simulink model "TosAeb"  (R2021a)
settings: Solver=FixedStepDiscrete  StartTime=0.0  StopTime=inf  FixedStep=0.01  RelTol=1e-3
undefined symbols (not defined in the file or in its folder): ACCModSts, AccSts1, AEB_ObjectMovingOrientation, AEB_ObjectMovingState, CN_BTCActive, CN_CurrentGearR, CN_CurrentGearR_DHT, CN_PTCActive, CN_VDCActive, CN_VSC_EspOff, CN_VSC_FCW_AEB_Error, CN_VSC_FCW_AEB_NoDispay, CN_VSC_FCW_AEB_PerfmDgdtn, CN_VSC_FCW_AEB_TempUnAvlbl, CN_VSC_VehStandstill, CNaccelAvoidMax, CNaccelAvoidMin, CNbrakePedalPress, CNcomfortStrLeftAccelMax, CNcomfortStrLeftAccelMin, CNcomfortStrRightAccelMax, CNcomfortStrRightAccelMin, CNDrvDoorOpen, CNDrvModeEco, CNDrvModeNorm, CNDrvModeOther, CNDrvModeSport, CNDrvSBRreminder, CNNull, CNobjInvalidID, CNPassDoorOpen, CNPassSBRreminder, CNPEBSAccelNegInf, CNPEBSAccelNull, CNPEBSTimeInf, CNPEBSTimeNull, CNSTOPMAX, CNSTOPNULL, CNsubjWidth, CNSwtOFF, CNSwtON, CNTTCMAX, CNTTCNULL, CNVehConfigAEBCycNone, CNVehConfigAEBNone, CNVehConfigAEBPedNone, CNVehConfigFCWNone, CNVehConfigJANone, CollisionSeverity, FALSE, FCWSnvty, JACycObjCalcInfoDefaultParams, JAPedObjCalcInfoDefaultParams, JAVehObjCalcInfoDefaultParams, latCycObjCalcInfoDefaultParams, latPedObjCalcInfoDefaultParams, longObjCalcInfoDefaultParams, ModSts, movingStatus, ObjectType ... (+1112)
libraries: simulink x3031, AEBSFunctionLib x50, Ta_Library x33, AEBSStateMachineLib x23, Mylibrary x12, simulink_extras x7, ObjSelect_lib x3, dspstat3 x2
(part 25 of 102)

== system TosAeb/FcwAebJa_Step_sys/ALG_AEBSystem/ALG_AEBController/AEB_FuncCondCalcApp/PedFunctionCondCalc/pedAEBfullSuppAborCond/pedAEBfullAborCondNoStandstillCalc ==
  inputSignals : Inport
  Bus Selector : BusSelector  OutputSignals=latPedTgtCalcInfo.latPedObjCalcInfo.latPedObjRawInfo.ObjectID,latPedTgtCalcInfo.latPedObjCalcInfo.latPedObjRawInfo.ObjectTypeCameraConfirmed,latPedTgtCalcInfo.latPedObjCalcInfo.CollisionRiskProb
  Bus Selector1 : BusSelector  OutputSignals=vehCanInfo.SteerWheelAngle,vehCanInfo.SteerWheelSpd,vehStateCalcInfo.VSCPedFuncSuppAborCond.VSCPedAEBfullAborCond,latFuncActiveStateTime.pedAEBfullWorkTime,vehStateCalcInfo.VSCVehStandStill
  Bus Selector2 : BusSelector  OutputSignals=latPedTgtCalcInfo.latPedCollsionAssesInfo.latCollisionSeverity,latPedTgtCalcInfo.latPedObjCalcInfo.CollisionRiskProb,latPedTgtCalcInfo.latPedObjCalcInfo.latPedObjRawInfo.dy,latPedTgtCalcInfo.latPedObjCalcInfo.latPedObjRawInfo.vy,latPedTgtCalcInfo.latPedObjCalcInfo.latPedObjRawInfo.dx,subjInfo.subjYawRate
  Ground : Ground
  pedAEBfullAborCondFinalJudge : Subsystem
  pedAEBfullObjRelActiveCondCalc : Subsystem
  pedAEBfullSituationRelAborCondCalc : Subsystem
  pedAEBfullVehRelAborCondCalc : Subsystem
  pedAEBfullAborCondNoStandstillFulfilled : Outport
  wires:
  Bus Selector2/6 -> pedAEBfullSituationRelAborCondCalc/7(subjYawRate)  "<subjYawRate>"
  Bus Selector2/5 -> pedAEBfullSituationRelAborCondCalc/6(dx)  "<dx>"
  Bus Selector/3 -> pedAEBfullObjRelActiveCondCalc/3(latCollsionRiskProb)  "<CollisionRiskProb>"
  Bus Selector/2 -> pedAEBfullObjRelActiveCondCalc/2(objIsFusionTarget)  "<ObjectTypeCameraConfirmed>"
  Bus Selector/1 -> pedAEBfullObjRelActiveCondCalc/1(ObjectID)  "<ObjectID>"
  Bus Selector2/4 -> pedAEBfullSituationRelAborCondCalc/5(vy)  "<vy>"
  Bus Selector2/3 -> pedAEBfullSituationRelAborCondCalc/4(dy)  "<dy>"
  Bus Selector2/2 -> pedAEBfullSituationRelAborCondCalc/3(objLatCollsionRiskProb)  "<CollisionRiskProb>"
  Bus Selector2/1 -> pedAEBfullSituationRelAborCondCalc/2(pedCollisionSeverity)  "<latCollisionSeverity>"
  Ground/1 -> pedAEBfullSituationRelAborCondCalc/1(pedVehVelReduced)
  Bus Selector1/5 -> pedAEBfullVehRelAborCondCalc/5(vehStateCalcStandstill)  "<VSCVehStandStill>"
  Bus Selector1/4 -> pedAEBfullVehRelAborCondCalc/4(pedAEBfullWorkTime)  "<pedAEBfullWorkTime>"
  Bus Selector1/3 -> pedAEBfullVehRelAborCondCalc/3(vehStateCalcAEBfullAborFlg)  "<VSCPedAEBfullAborCond>"
  Bus Selector1/2 -> pedAEBfullVehRelAborCondCalc/2(SteerWheelSpd)  "<SteerWheelSpd>"
  Bus Selector1/1 -> pedAEBfullVehRelAborCondCalc/1(SteerWheelAngle)  "<SteerWheelAngle>"
  inputSignals/1 -> Bus Selector1/1, Bus Selector2/1, Bus Selector/1
  pedAEBfullAborCondFinalJudge/1(pedAEBfullAborCondNoStandstillFulfilled) -> pedAEBfullAborCondNoStandstillFulfilled/1
  pedAEBfullSituationRelAborCondCalc/1(pedAEBfullSituationRelAborCondFulfilled) -> pedAEBfullAborCondFinalJudge/3(pedAEBfullSituationRelAborCondFulfilled)  "AEB_pedAEBfullSitRelAborCond"
  pedAEBfullVehRelAborCondCalc/1(pedAEBfullVehRelAborCondFulfilled) -> pedAEBfullAborCondFinalJudge/2(pedAEBfullVehRelAborCondFulfilled)  "AEB_pedAEBfullVehRelAborCond"
  pedAEBfullObjRelActiveCondCalc/1(pedAEBfullObjRelAborCondFulfilled) -> pedAEBfullAborCondFinalJudge/1(pedAEBfullObjRelAborCondFulfilled)  "AEB_pedAEBfullObjRelAborCond"

== system TosAeb/FcwAebJa_Step_sys/ALG_AEBSystem/ALG_AEBController/AEB_FuncCondCalcApp/PedFunctionCondCalc/pedAEBfullSuppAborCond/pedAEBfullAborCondStandstillCalc ==
  inputSignals : Inport
  Bus Selector : BusSelector  OutputSignals=subjInfo.subjVel,vehCanInfo.SteerWheelAngle,vehCanInfo.SteerWheelSpd,vehStateCalcInfo.VSCPedFuncSuppAborCond.VSCPedAEBfullAborCond,latFuncActiveStateTime.pedAEBfullWorkTime,vehStateCalcInfo.VSCVehStandStill
  pedAEBfullVehRelAborCondCalc : Subsystem
  pedAEBfullAborCondStandstillFulfilled : Outport
  wires:
  Bus Selector/6 -> pedAEBfullVehRelAborCondCalc/6(vehStateCalcStandstill)  "<VSCVehStandStill>"
  Bus Selector/5 -> pedAEBfullVehRelAborCondCalc/5(pedAEBfullWorkTime)  "<pedAEBfullWorkTime>"
  Bus Selector/1 -> pedAEBfullVehRelAborCondCalc/1(subjVel)  "<subjVel>"
  Bus Selector/4 -> pedAEBfullVehRelAborCondCalc/4(vehStateCalcAEBfullAborFlg)  "<VSCPedAEBfullAborCond>"
  Bus Selector/2 -> pedAEBfullVehRelAborCondCalc/2(SteerWheelAngle)  "<SteerWheelAngle>"
  Bus Selector/3 -> pedAEBfullVehRelAborCondCalc/3(SteerWheelSpd)  "<SteerWheelSpd>"
  inputSignals/1 -> Bus Selector/1
  pedAEBfullVehRelAborCondCalc/1(pedAEBfullVehRelAborCondFulfilled) -> pedAEBfullAborCondStandstillFulfilled/1

== system TosAeb/FcwAebJa_Step_sys/ALG_AEBSystem/ALG_AEBController/AEB_FuncCondCalcApp/PedFunctionCondCalc/pedAEBfullSuppAborCond/pedAEBfullSuppCondCalc ==
  inputSignals : Inport
  Bus Selector : BusSelector  OutputSignals=latPedTgtCalcInfo.latPedObjCalcInfo.latPedObjRawInfo.ObjectID,latPedTgtCalcInfo.latPedObjCalcInfo.latPedObjAbsInfo.vxAbsObj,latPedTgtCalcInfo.latPedObjCalcInfo.latPedObjAbsInfo.ObjFusQuality,latPedTgtCalcInfo.latPedObjCalcInfo.latPedObjRawInfo.dy,latPedTgtCalcInfo.latPedObjCalcInfo.latPedObjRawInfo.vy
  Bus Selector1 : BusSelector  OutputSignals=subjInfo.subjVel,vehConfigInfo.VehConfigIbooster,vehCanInfo.ESP_MasterCylindBrakePressValid,vehCanInfo.ESP_MasterCylindBrakePress,vehCanInfo.SteerWheelAngle,vehCanInfo.SteerWheelSpd,vehCanInfo.AccrPedlPosnDiagc,vehStateCalcInfo.VSCPedFuncSuppAborCond.VSCPedAEBfullSuppCond
  Bus Selector2 : BusSelector  OutputSignals=latPedTgtCalcInfo.latPedCollsionAssesInfo.latCollisionSeverity,subjInfo.subjCurvTraj,subjInfo.subjLatAccel,latPedTgtCalcInfo.latPedObjCalcInfo.PreCollisionFlg,latPedTgtCalcInfo.latPedObjCalcInfo.CollisionRiskProb,latPedTgtCalcInfo.latPedObjCalcInfo.latPedObjRawInfo.dy,vehStateCalcInfo.VSC_AEBFuncSwtSts.FCWSnvty,subjInfo.subjVel,latPedTgtCalcInfo.latPedObjCalcInfo.latPedObjAbsInfo.dxFrontBumper,latPedTgtCalcInfo.latPedObjCalcInfo.latPedObjRawInfo.ObjectTypeCameraConfirmed,latPedTgtCalcInfo.latPedCollsionAssesInfo.distSecure,latPedTgtCalcInfo.latPedCollsionAssesInfo.distBraking,latPedTgtCalcInfo.latPedObjCalcInfo.latPedObjRawInfo.vy
  pedAEBfullObjRelSuppCondCalc : Subsystem
  pedAEBfullSituationRelSuppCondCalc : Subsystem
  pedAEBfullSuppCondFinalJudge : Subsystem
  pedAEBfullVehRelSuppCondCalc : Subsystem
  pedAEBfullSuppCondFulfilled : Outport
  wires:
  Bus Selector1/2 -> pedAEBfullVehRelSuppCondCalc/2(VehConfigIbooster)  "<VehConfigIbooster>"
  Bus Selector/5 -> pedAEBfullObjRelSuppCondCalc/5(vy)  "<vy>"
  Bus Selector/4 -> pedAEBfullObjRelSuppCondCalc/4(dy)  "<dy>"
  Bus Selector/3 -> pedAEBfullObjRelSuppCondCalc/3(ObjFusQuality)  "<ObjFusQuality>"
  Bus Selector2/13 -> pedAEBfullSituationRelSuppCondCalc/13(vy)  "<vy>"
  Bus Selector/2 -> pedAEBfullObjRelSuppCondCalc/2(vxAbsObj)  "<vxAbsObj>"
  Bus Selector2/12 -> pedAEBfullSituationRelSuppCondCalc/12(distBraking)  "<distBraking>"
  Bus Selector2/11 -> pedAEBfullSituationRelSuppCondCalc/11(distSecure)  "<distSecure>"
  Bus Selector2/10 -> pedAEBfullSituationRelSuppCondCalc/10(ObjectTypeCameraConfirmed)  "<ObjectTypeCameraConfirmed>"
  Bus Selector2/9 -> pedAEBfullSituationRelSuppCondCalc/9(dxFrontBumper)  "<dxFrontBumper>"
  Bus Selector2/8 -> pedAEBfullSituationRelSuppCondCalc/8(subjVel)  "<subjVel>"
  Bus Selector2/7 -> pedAEBfullSituationRelSuppCondCalc/7(FcwHMISetting)  "<FCWSnvty>"
  Bus Selector2/6 -> pedAEBfullSituationRelSuppCondCalc/6(dy)  "<dy>"
  Bus Selector2/5 -> pedAEBfullSituationRelSuppCondCalc/5(pedCollisionRisk)  "<CollisionRiskProb>"
  Bus Selector2/4 -> pedAEBfullSituationRelSuppCondCalc/4(pedCollisionProbFlg)  "<PreCollisionFlg>"
  Bus Selector2/3 -> pedAEBfullSituationRelSuppCondCalc/3(subjLatAccel)  "<subjLatAccel>"
  Bus Selector2/1 -> pedAEBfullSituationRelSuppCondCalc/1(latCollisionSeverity)  "<latCollisionSeverity>"
  Bus Selector2/2 -> pedAEBfullSituationRelSuppCondCalc/2(subjCurvTraj)  "<subjCurvTraj>"
  Bus Selector1/8 -> pedAEBfullVehRelSuppCondCalc/8(vehStateCalcPedAEBfullSuppFlg)  "<VSCPedAEBfullSuppCond>"
  Bus Selector1/7 -> pedAEBfullVehRelSuppCondCalc/7(AccrPedlPosnDiagc)  "<AccrPedlPosnDiagc>"
  Bus Selector1/6 -> pedAEBfullVehRelSuppCondCalc/6(SteerWheelSpd)  "<SteerWheelSpd>"
  Bus Selector1/5 -> pedAEBfullVehRelSuppCondCalc/5(SteerWheelAngle)  "<SteerWheelAngle>"
  Bus Selector1/4 -> pedAEBfullVehRelSuppCondCalc/4(ESP_MasterCylindBrakePress)  "<ESP_MasterCylindBrakePress>"
  Bus Selector1/3 -> pedAEBfullVehRelSuppCondCalc/3(ESP_MasterCylindBrakePressValid)  "<ESP_MasterCylindBrakePressValid>"
  Bus Selector1/1 -> pedAEBfullVehRelSuppCondCalc/1(subjVel)  "<subjVel>"
  Bus Selector/1 -> pedAEBfullObjRelSuppCondCalc/1(ObjectID)  "<ObjectID>"
  inputSignals/1 -> Bus Selector/1, Bus Selector1/1, Bus Selector2/1
  pedAEBfullSituationRelSuppCondCalc/1(pedAEBfullSituationRelSuppCondFulfilled) -> pedAEBfullSuppCondFinalJudge/3(pedAEBfullSituationRelSuppCondFulfilled)  "AEB_pedAEBfullSitRelSuppCond"
  pedAEBfullObjRelSuppCondCalc/1(pedAEBfullObjRelSuppCondFulfilled) -> pedAEBfullSuppCondFinalJudge/1(pedAEBfullObjRelSuppCondFulfilled)  "AEB_pedAEBfullObjRelSuppCond"
  pedAEBfullVehRelSuppCondCalc/1(pedAEBfullVehRelSuppCondFulfilled) -> pedAEBfullSuppCondFinalJudge/2(pedAEBfullVehRelSuppCondFulfilled)  "AEB_pedAEBfullVehRelSuppCond"
  pedAEBfullSuppCondFinalJudge/1(pedAEBfullSuppCondFulfilled) -> pedAEBfullSuppCondFulfilled/1

== system TosAeb/FcwAebJa_Step_sys/ALG_AEBSystem/ALG_AEBController/AEB_FuncCondCalcApp/PedFunctionCondCalc/pedPreWarningSuppAborCond/pedPreWarningAborCondCalc ==
  inputSignals : Inport
  Bus Selector : BusSelector  OutputSignals=latPedTgtCalcInfo.latPedObjCalcInfo.latPedObjRawInfo.ObjectID,latFuncActiveStateTime.pedPreWarningWorkTime
  Bus Selector1 : BusSelector  OutputSignals=vehStateCalcInfo.VSCPedFuncSuppAborCond.VSCPedPreWarningSuppCond,latFuncActiveStateTime.pedPreWarningWorkTime
  Bus Selector2 : BusSelector  OutputSignals=latPedTgtCalcInfo.latPedCollsionAssesInfo.latCollisionSeverity,latFuncActiveStateTime.pedPreWarningWorkTime
  pedPreWarningAborCondFinalJudge : Subsystem
  pedPreWarningObjRelActiveCondCalc : Subsystem
  pedPreWarningSituationRelAborCondCalc : Subsystem
  pedPreWarningVehRelAborCondCalc : Subsystem
  pedPreWarningAborCondFulfilled : Outport
  wires:
  Bus Selector2/2 -> pedPreWarningSituationRelAborCondCalc/2(pedPreWarningWorkTime)  "<pedPreWarningWorkTime>"
  Bus Selector2/1 -> pedPreWarningSituationRelAborCondCalc/1(latCollisionSeverity)  "<latCollisionSeverity>"
  Bus Selector1/2 -> pedPreWarningVehRelAborCondCalc/2(pedPreWarningWorkTime)  "<pedPreWarningWorkTime>"
  Bus Selector1/1 -> pedPreWarningVehRelAborCondCalc/1(vehStateCalcPreWarningAborFlg)  "<VSCPedPreWarningSuppCond>"
  Bus Selector/2 -> pedPreWarningObjRelActiveCondCalc/2(pedPreWarningWorkTime)  "<pedPreWarningWorkTime>"
  inputSignals/1 -> Bus Selector1/1, Bus Selector2/1, Bus Selector/1
  Bus Selector/1 -> pedPreWarningObjRelActiveCondCalc/1(ObjectID)  "<ObjectID>"
  pedPreWarningObjRelActiveCondCalc/1(pedPreWarningObjRelAborCondFulfilled) -> pedPreWarningAborCondFinalJudge/1(pedPreWarningObjRelAborCondFulfilled)  "AEB_pedPreWarningObjRelAborCond"
  pedPreWarningVehRelAborCondCalc/1(pedPreWarningVehRelAborCondFulfilled) -> pedPreWarningAborCondFinalJudge/2(pedPreWarningVehRelAborCondFulfilled)  "AEB_pedPreWarningVehRelAborCond"
  pedPreWarningSituationRelAborCondCalc/1(pedPreWarningSituationRelAborCondFulfilled) -> pedPreWarningAborCondFinalJudge/3(pedPreWarningSituationRelAborCondFulfilled)  "AEB_pedPreWarningSitRelAborCond"
  pedPreWarningAborCondFinalJudge/1(pedPreWarningAborCondFulfilled) -> pedPreWarningAborCondFulfilled/1

== system TosAeb/FcwAebJa_Step_sys/ALG_AEBSystem/ALG_AEBController/AEB_FuncCondCalcApp/PedFunctionCondCalc/pedPreWarningSuppAborCond/pedPreWarningSuppCondCalc ==
  inputSignals : Inport
  Bus Selector : BusSelector  OutputSignals=latPedTgtCalcInfo.latPedObjCalcInfo.latPedObjRawInfo.ObjectID,latPedTgtCalcInfo.latPedObjCalcInfo.latPedObjAbsInfo.vxAbsObj,latPedTgtCalcInfo.latPedObjCalcInfo.latPedObjAbsInfo.ObjFusQuality,latPedTgtCalcInfo.latPedObjCalcInfo.latPedObjRawInfo.dy,latPedTgtCalcInfo.latPedObjCalcInfo.latPedObjRawInfo.vy
  Bus Selector1 : BusSelector  OutputSignals=subjInfo.subjVel,vehConfigInfo.VehConfigIbooster,vehCanInfo.ESP_MasterCylindBrakePressValid,vehCanInfo.ESP_MasterCylindBrakePress,vehCanInfo.IB_BrkPedalAppld,vehCanInfo.SteerWheelAngle,vehCanInfo.SteerWheelSpd,vehCanInfo.AccrPedlPosnDiagc,vehStateCalcInfo.VSCPedFuncSuppAborCond.VSCPedPreWarningAborCond
  Bus Selector2 : BusSelector  OutputSignals=latPedTgtCalcInfo.latPedCollsionAssesInfo.latCollisionSeverity,subjInfo.subjCurvTraj,subjInfo.subjLatAccel,latPedTgtCalcInfo.latPedObjCalcInfo.latPedObjRawInfo.ObjectTypeCameraConfirmed,latPedTgtCalcInfo.latPedObjCalcInfo.CollisionRiskProb,latPedTgtCalcInfo.latPedObjCalcInfo.latPedObjRawInfo.vy,latPedTgtCalcInfo.latPedObjCalcInfo.latPedObjAbsInfo.dxFrontBumper,vehStateCalcInfo.VSC_AEBFuncSwtSts.FCWSnvty,subjInfo.subjVel
  pedPreWarningObjRelActiveCondCalc : Subsystem
  pedPreWarningSituationRelActiveCondCalc : Subsystem
  pedPreWarningSuppCondFinalJudge : Subsystem
  pedPreWarningVehRelActiveCondCalc : Subsystem
  pedPreWarningSuppCondFulfilled : Outport
  wires:
  Bus Selector1/2 -> pedPreWarningVehRelActiveCondCalc/2(VehConfigIbooster)  "<VehConfigIbooster>"
  Bus Selector/5 -> pedPreWarningObjRelActiveCondCalc/5(vy)  "<vy>"
  Bus Selector/4 -> pedPreWarningObjRelActiveCondCalc/4(dy)  "<dy>"
  Bus Selector/3 -> pedPreWarningObjRelActiveCondCalc/3(ObjFusQuality)  "<ObjFusQuality>"
  Bus Selector/2 -> pedPreWarningObjRelActiveCondCalc/2(vxAbsObj)  "<vxAbsObj>"
  Bus Selector1/5 -> pedPreWarningVehRelActiveCondCalc/5(IB_BrkPedalAppld)  "<IB_BrkPedalAppld>"
  Bus Selector2/9 -> pedPreWarningSituationRelActiveCondCalc/9(subjVel)  "<subjVel>"
  Bus Selector2/8 -> pedPreWarningSituationRelActiveCondCalc/8(FcwHMISetting)  "<FCWSnvty>"
  Bus Selector2/7 -> pedPreWarningSituationRelActiveCondCalc/7(dxFrontBumper)  "<dxFrontBumper>"
  Bus Selector2/6 -> pedPreWarningSituationRelActiveCondCalc/6(vy)  "<vy>"
  Bus Selector2/5 -> pedPreWarningSituationRelActiveCondCalc/5(colliisonRiskProb)  "<CollisionRiskProb>"
  Bus Selector2/4 -> pedPreWarningSituationRelActiveCondCalc/4(ObjectTypeCameraConfirmed)  "<ObjectTypeCameraConfirmed>"
  Bus Selector2/3 -> pedPreWarningSituationRelActiveCondCalc/3(subjLatAccel)  "<subjLatAccel>"
  Bus Selector2/2 -> pedPreWarningSituationRelActiveCondCalc/2(subjCurvTraj)  "<subjCurvTraj>"
  Bus Selector2/1 -> pedPreWarningSituationRelActiveCondCalc/1(latCollisionSeverity)  "<latCollisionSeverity>"
  inputSignals/1 -> Bus Selector/1, Bus Selector1/1, Bus Selector2/1
  Bus Selector1/9 -> pedPreWarningVehRelActiveCondCalc/9(vehStateCalcPedPreWarningSuppFlg)  "<VSCPedPreWarningAborCond>"
  Bus Selector1/1 -> pedPreWarningVehRelActiveCondCalc/1(subjVel)  "<subjVel>"
  Bus Selector1/8 -> pedPreWarningVehRelActiveCondCalc/8(AccrPedlPosnDiagc)  "<AccrPedlPosnDiagc>"
  Bus Selector1/3 -> pedPreWarningVehRelActiveCondCalc/3(ESP_MasterCylindBrakePressValid)  "<ESP_MasterCylindBrakePressValid>"
  Bus Selector1/7 -> pedPreWarningVehRelActiveCondCalc/7(SteerWheelSpd)  "<SteerWheelSpd>"
  Bus Selector1/4 -> pedPreWarningVehRelActiveCondCalc/4(ESP_MasterCylindBrakePress)  "<ESP_MasterCylindBrakePress>"
  Bus Selector1/6 -> pedPreWarningVehRelActiveCondCalc/6(SteerWheelAngle)  "<SteerWheelAngle>"
  Bus Selector/1 -> pedPreWarningObjRelActiveCondCalc/1(ObjectID)  "<ObjectID>"
  pedPreWarningSuppCondFinalJudge/1(pedPreWarningSuppCondFulfilled) -> pedPreWarningSuppCondFulfilled/1
  pedPreWarningVehRelActiveCondCalc/1(pedPreWarningVehRelSuppCondFulfilled) -> pedPreWarningSuppCondFinalJudge/2(pedPreWarningVehRelSuppCondFulfilled)  "AEB_pedPreWarningVehRelSuppCond"
  pedPreWarningObjRelActiveCondCalc/1(pedPreWarningObjRelSuppCondFulfilled) -> pedPreWarningSuppCondFinalJudge/1(pedPreWarningObjRelSuppCondFulfilled)  "AEB_pedPreWarningObjRelSuppCond"
  pedPreWarningSituationRelActiveCondCalc/1(pedPreWarningSituationRelSuppCondFulfilled) -> pedPreWarningSuppCondFinalJudge/3(pedPreWarningSituationRelSuppCondFulfilled)  "AEB_pedPreWarningSitRelSuppCond"

== system TosAeb/FcwAebJa_Step_sys/ALG_AEBSystem/ALG_AEBController/AEB_FuncCondCalcApp/PedFunctionCondCalc/pedPrefillSuppAborCond/pedPrefillAborCondCalc ==
  inputSignals : Inport
  Bus Selector : BusSelector  OutputSignals=latPedTgtCalcInfo.latPedObjCalcInfo.latPedObjRawInfo.ObjectID
  Bus Selector1 : BusSelector  OutputSignals=vehCanInfo.SteerWheelAngle,vehCanInfo.SteerWheelSpd,vehStateCalcInfo.VSCPedFuncSuppAborCond.VSCPedPrefillAborCond,latFuncActiveStateTime.pedPrefillWorkTime
  Bus Selector2 : BusSelector  OutputSignals=latPedTgtCalcInfo.latPedCollsionAssesInfo.latCollisionSeverity,latFuncActiveStateTime.pedPrefillWorkTime
  pedPrefillAborCondFinalJudge : Subsystem
  pedPrefillObjRelAborCondCalc : Subsystem
  pedPrefillSituationRelAborCondCalc : Subsystem
  pedPrefillVehRelAborCondCalc : Subsystem
  pedPrefillAborCondFulfilled : Outport
  wires:
  Bus Selector2/2 -> pedPrefillSituationRelAborCondCalc/2(pedPrefillWorkTime)  "<pedPrefillWorkTime>"
  Bus Selector2/1 -> pedPrefillSituationRelAborCondCalc/1(PedCollisionSeverity)  "<latCollisionSeverity>"
  Bus Selector1/1 -> pedPrefillVehRelAborCondCalc/1(SteerWheelAngle)  "<SteerWheelAngle>"
  Bus Selector1/2 -> pedPrefillVehRelAborCondCalc/2(SteerWheelSpd)  "<SteerWheelSpd>"
  Bus Selector1/3 -> pedPrefillVehRelAborCondCalc/3(vehStateCalcPedPrefillAborFlg)  "<VSCPedPrefillAborCond>"
  Bus Selector1/4 -> pedPrefillVehRelAborCondCalc/4(pedPrefillWorkTime)  "<pedPrefillWorkTime>"
  Bus Selector/1 -> pedPrefillObjRelAborCondCalc/1(ObjectID)  "<ObjectID>"
  inputSignals/1 -> Bus Selector/1, Bus Selector1/1, Bus Selector2/1
  pedPrefillObjRelAborCondCalc/1(pedPrefillObjRelAborCondFulfilled) -> pedPrefillAborCondFinalJudge/1(pedPrefillObjRelAborCondFulfilled)  "AEB_pedPrefillObjRelAborCond"
  pedPrefillVehRelAborCondCalc/1(pedPrefillVehRelAborCondFulfilled) -> pedPrefillAborCondFinalJudge/2(pedPrefillVehRelAborCondFulfilled)  "AEB_pedPrefillVehRelAborCond"
  pedPrefillSituationRelAborCondCalc/1(pedPrefillSituationRelAborCondFulfilled) -> pedPrefillAborCondFinalJudge/3(pedPrefillSituationRelAborCondFulfilled)  "AEB_pedPrefillSitRelAborCond"
  pedPrefillAborCondFinalJudge/1(pedPrefillAborCondFulfilled) -> pedPrefillAborCondFulfilled/1

== system TosAeb/FcwAebJa_Step_sys/ALG_AEBSystem/ALG_AEBController/AEB_FuncCondCalcApp/PedFunctionCondCalc/pedPrefillSuppAborCond/pedPrefillSuppCondCalc ==
  inputSignals : Inport
  Bus Selector : BusSelector  OutputSignals=latPedTgtCalcInfo.latPedObjCalcInfo.latPedObjRawInfo.ObjectID,latPedTgtCalcInfo.latPedObjCalcInfo.latPedObjAbsInfo.vxAbsObj,latPedTgtCalcInfo.latPedObjCalcInfo.latPedObjAbsInfo.ObjFusQuality,latPedTgtCalcInfo.latPedObjCalcInfo.latPedObjRawInfo.dy,latPedTgtCalcInfo.latPedObjCalcInfo.latPedObjRawInfo.vy
  Bus Selector1 : BusSelector  OutputSignals=subjInfo.subjVel,vehConfigInfo.VehConfigIbooster,vehCanInfo.ESP_MasterCylindBrakePressValid,vehCanInfo.ESP_MasterCylindBrakePress,vehCanInfo.IB_BrkPedalAppld,vehCanInfo.SteerWheelAngle,vehCanInfo.SteerWheelSpd,vehCanInfo.AccrPedlPosnDiagc,vehStateCalcInfo.VSCVehSuppAborCond.VSCvehPrefillSuppCond
  Bus Selector2 : BusSelector  OutputSignals=latPedTgtCalcInfo.latPedCollsionAssesInfo.latCollisionSeverity,subjInfo.subjCurvTraj,subjInfo.subjLatAccel,latPedTgtCalcInfo.latPedObjCalcInfo.CollisionRiskProb,latPedTgtCalcInfo.latPedObjCalcInfo.latPedObjRawInfo.ObjectTypeCameraConfirmed,latPedTgtCalcInfo.latPedObjCalcInfo.latPedObjRawInfo.dy,latPedTgtCalcInfo.latPedObjCalcInfo.latPedObjRawInfo.vy,latPedTgtCalcInfo.latPedObjCalcInfo.latPedObjAbsInfo.dxFrontBumper,latPedTgtCalcInfo.latPedCollsionAssesInfo.distBraking,latPedTgtCalcInfo.latPedCollsionAssesInfo.distSecure,subjInfo.subjVel
  pedPrefillObjRelActiveCondCalc : Subsystem
  pedPrefillSituationRelActiveCondCalc : Subsystem
  pedPrefillSuppCondFinalJudge : Subsystem
  pedPrefillVehRelActiveCondCalc : Subsystem
  pedPrefillSuppCondFulfilled : Outport
  wires:
  Bus Selector1/2 -> pedPrefillVehRelActiveCondCalc/2(VehConfigIbooster)  "<VehConfigIbooster>"
  Bus Selector/5 -> pedPrefillObjRelActiveCondCalc/5(vy)  "<vy>"
  Bus Selector/4 -> pedPrefillObjRelActiveCondCalc/4(dy)  "<dy>"
  Bus Selector/3 -> pedPrefillObjRelActiveCondCalc/3(ObjFusQuality)  "<ObjFusQuality>"
  Bus Selector/2 -> pedPrefillObjRelActiveCondCalc/2(vxAbsObj)  "<vxAbsObj>"
  Bus Selector1/5 -> pedPrefillVehRelActiveCondCalc/5(IB_BrkPedalAppld)  "<IB_BrkPedalAppld>"
  Bus Selector2/11 -> pedPrefillSituationRelActiveCondCalc/11(subVel)  "<subjVel>"
  Bus Selector2/10 -> pedPrefillSituationRelActiveCondCalc/10(distSecure)  "<distSecure>"
  Bus Selector2/9 -> pedPrefillSituationRelActiveCondCalc/9(longDist4Brk)  "<distBraking>"
  Bus Selector2/8 -> pedPrefillSituationRelActiveCondCalc/8(dxFrontBumper)  "<dxFrontBumper>"
  Bus Selector2/7 -> pedPrefillSituationRelActiveCondCalc/7(vy)  "<vy>"
  Bus Selector2/6 -> pedPrefillSituationRelActiveCondCalc/6(dy)  "<dy>"
  Bus Selector2/5 -> pedPrefillSituationRelActiveCondCalc/5(ObjectTypeCameraConfirmed)  "<ObjectTypeCameraConfirmed>"
  Bus Selector2/4 -> pedPrefillSituationRelActiveCondCalc/4(colliisonRiskProb)  "<CollisionRiskProb>"
  Bus Selector2/3 -> pedPrefillSituationRelActiveCondCalc/3(subjLatAccel)  "<subjLatAccel>"
  Bus Selector2/2 -> pedPrefillSituationRelActiveCondCalc/2(subjCurvTraj)  "<subjCurvTraj>"
  Bus Selector2/1 -> pedPrefillSituationRelActiveCondCalc/1(latCollisionSeverity)  "<latCollisionSeverity>"
  Bus Selector1/1 -> pedPrefillVehRelActiveCondCalc/1(subjVel)  "<subjVel>"
  Bus Selector1/9 -> pedPrefillVehRelActiveCondCalc/9(vehStateCalcpedPrefillSuppFlg)  "<VSCvehPrefillSuppCond>"
  Bus Selector1/3 -> pedPrefillVehRelActiveCondCalc/3(ESP_MasterCylindBrakePressValid)  "<ESP_MasterCylindBrakePressValid>"
  Bus Selector1/4 -> pedPrefillVehRelActiveCondCalc/4(ESP_MasterCylindBrakePress)  "<ESP_MasterCylindBrakePress>"
  Bus Selector1/6 -> pedPrefillVehRelActiveCondCalc/6(SteerWheelAngle)  "<SteerWheelAngle>"
  Bus Selector1/8 -> pedPrefillVehRelActiveCondCalc/8(AccrPedlPosnDiagc)  "<AccrPedlPosnDiagc>"
  Bus Selector1/7 -> pedPrefillVehRelActiveCondCalc/7(SteerWheelSpd)  "<SteerWheelSpd>"
  Bus Selector/1 -> pedPrefillObjRelActiveCondCalc/1(ObjectID)  "<ObjectID>"
  inputSignals/1 -> Bus Selector/1, Bus Selector1/1, Bus Selector2/1
  pedPrefillSuppCondFinalJudge/1(pedPrefillSuppCondFulfilled) -> pedPrefillSuppCondFulfilled/1
  pedPrefillVehRelActiveCondCalc/1(pedPrefillVehRelSuppCondFulfilled) -> pedPrefillSuppCondFinalJudge/2(pedPrefillVehRelSuppCondFulfilled)  "AEB_pedPrefillVehRelSuppCond"
  pedPrefillObjRelActiveCondCalc/1(pedPrefillObjRelSuppCondFulfilled) -> pedPrefillSuppCondFinalJudge/1(pedPrefillObjRelSuppCondFulfilled)  "AEB_pedPrefillObjRelSuppCond"
  pedPrefillSituationRelActiveCondCalc/1(pedPrefillSituationRelSuppCondFulfilled) -> pedPrefillSuppCondFinalJudge/3(pedPrefillSituationRelSuppCondFulfilled)  "AEB_pedPrefillSitRelSuppCond"

== system TosAeb/FcwAebJa_Step_sys/ALG_AEBSystem/ALG_AEBController/AEB_FuncImpManagementApp/FuncBlockTimeCalc/tBlockTimeBrkCalc/BlockTimeCalc ==
  blockTimeTriggerCond : Inport
  BlockTimeCalc : Stateflow chart  TreatAsAtomicUnit=on
  Constant : Constant  Value=Ts  OutDataTypeStr=single
  Constant1 : Constant  Value=P_AEB_FIM_BrkBlockTimeThres  OutDataTypeStr=single
  blockTime : Outport
  wires:
  Constant1/1 -> BlockTimeCalc/3(PblockTime)
  Constant/1 -> BlockTimeCalc/2(Ts)
  blockTimeTriggerCond/1 -> BlockTimeCalc/1(blockTimeTriggerCond)
  BlockTimeCalc/1(blockTime) -> blockTime/1

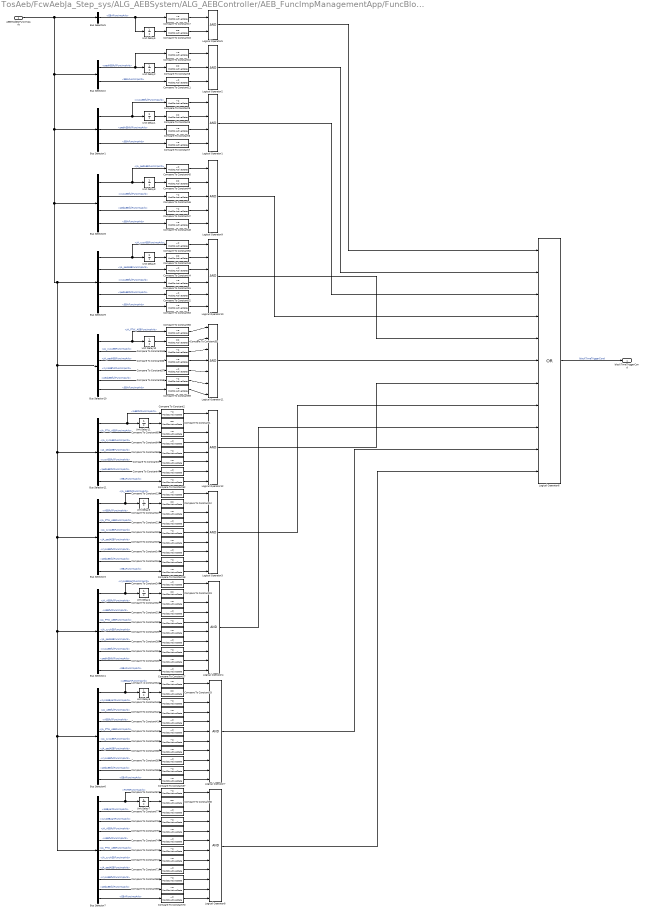
== system TosAeb/FcwAebJa_Step_sys/ALG_AEBSystem/ALG_AEBController/AEB_FuncImpManagementApp/FuncBlockTimeCalc/tBlockTimeBrkCalc/blockTimeTriggerCondCalc ==
  AEBModStsFuncImpArb : Inport  OutDataTypeStr=Bus: BusAEBModStsFuncImpArb
  Bus Selector1 : BusSelector  OutputSignals=brkFuncImpArb.cycAEBfullFuncImpArb,brkFuncImpArb.pedAEBfullFuncImpArb,brkFuncImpArb.EBAFuncImpArb
  Bus Selector10 : BusSelector  OutputSignals=brkFuncImpArb.JA_PTW_AEBFuncImpArb,brkFuncImpArb.JA_cycAEBFuncImpArb,brkFuncImpArb.JA_pedAEBFuncImpArb,brkFuncImpArb.cycAEBfullFuncImpArb,brkFuncImpArb.pedAEBfullFuncImpArb,brkFuncImpArb.EBAFuncImpArb
  Bus Selector11 : BusSelector  OutputSignals=brkFuncImpArb.AEBfullFuncImpArb,brkFuncImpArb.JA_PTW_AEBFuncImpArb,brkFuncImpArb.JA_cycAEBFuncImpArb,brkFuncImpArb.JA_pedAEBFuncImpArb,brkFuncImpArb.cycAEBfullFuncImpArb,brkFuncImpArb.pedAEBfullFuncImpArb,brkFuncImpArb.EBAFuncImpArb
  Bus Selector2 : BusSelector  OutputSignals=brkFuncImpArb.pedAEBfullFuncImpArb,brkFuncImpArb.EBAFuncImpArb
  Bus Selector3 : BusSelector  OutputSignals=brkFuncImpArb.JA_AEBfullFuncImpArb,brkFuncImpArb.AEBfullFuncImpArb,brkFuncImpArb.JA_PTW_AEBFuncImpArb,brkFuncImpArb.JA_cycAEBFuncImpArb,brkFuncImpArb.JA_pedAEBFuncImpArb,brkFuncImpArb.cycAEBfullFuncImpArb,brkFuncImpArb.pedAEBfullFuncImpArb,brkFuncImpArb.EBAFuncImpArb
  Bus Selector4 : BusSelector  OutputSignals=brkFuncImpArb.cycAEBpartFuncImpArb,brkFuncImpArb.JA_AEBfullFuncImpArb,brkFuncImpArb.AEBfullFuncImpArb,brkFuncImpArb.JA_PTW_AEBFuncImpArb,brkFuncImpArb.JA_cycAEBFuncImpArb,brkFuncImpArb.JA_pedAEBFuncImpArb,brkFuncImpArb.cycAEBfullFuncImpArb,brkFuncImpArb.pedAEBfullFuncImpArb,brkFuncImpArb.EBAFuncImpArb
  Bus Selector5 : BusSelector  OutputSignals=brkFuncImpArb.EBAFuncImpArb
  Bus Selector6 : BusSelector  OutputSignals=brkFuncImpArb.AEBpartFuncImpArb,brkFuncImpArb.cycAEBpartFuncImpArb,brkFuncImpArb.JA_AEBfullFuncImpArb,brkFuncImpArb.AEBfullFuncImpArb,brkFuncImpArb.JA_PTW_AEBFuncImpArb,brkFuncImpArb.JA_cycAEBFuncImpArb,brkFuncImpArb.JA_pedAEBFuncImpArb,brkFuncImpArb.cycAEBfullFuncImpArb,brkFuncImpArb.pedAEBfullFuncImpArb,brkFuncImpArb.EBAFuncImpArb
  Bus Selector7 : BusSelector  OutputSignals=brkFuncImpArb.FCTBFuncImpArb,brkFuncImpArb.AEBpartFuncImpArb,brkFuncImpArb.cycAEBpartFuncImpArb,brkFuncImpArb.JA_AEBfullFuncImpArb,brkFuncImpArb.AEBfullFuncImpArb,brkFuncImpArb.JA_PTW_AEBFuncImpArb,brkFuncImpArb.JA_cycAEBFuncImpArb,brkFuncImpArb.JA_pedAEBFuncImpArb,brkFuncImpArb.cycAEBfullFuncImpArb,brkFuncImpArb.pedAEBfullFuncImpArb,brkFuncImpArb.EBAFuncImpArb
  Bus Selector8 : BusSelector  OutputSignals=brkFuncImpArb.JA_pedAEBFuncImpArb,brkFuncImpArb.cycAEBfullFuncImpArb,brkFuncImpArb.pedAEBfullFuncImpArb,brkFuncImpArb.EBAFuncImpArb
  Bus Selector9 : BusSelector  OutputSignals=brkFuncImpArb.JA_cycAEBFuncImpArb,brkFuncImpArb.JA_pedAEBFuncImpArb,brkFuncImpArb.cycAEBfullFuncImpArb,brkFuncImpArb.pedAEBfullFuncImpArb,brkFuncImpArb.EBAFuncImpArb
  Compare To Constant{1,5,8,12,16,23,33,44,49,55,66} (11 alike) : simulink/Logic and Bit Operations/Compare To Constant  relop===  const=ModSts.ActiveState  OutDataTypeStr=boolean
  Compare To Constant{2..4,6..7,9..11,13..15,17..22,24..32,34..43,45..48,50..54,56..65,67..77} (66 alike) : simulink/Logic and Bit Operations/Compare To Constant  relop=~=  const=ModSts.ActiveState  OutDataTypeStr=boolean
  Logical Operator1 : Logic  Inputs=4  AllPortsSameDT=off  OutDataTypeStr=boolean
  Logical Operator10 : Logic  Inputs=6  AllPortsSameDT=off  OutDataTypeStr=boolean
  Logical Operator11 : Logic  Inputs=7  AllPortsSameDT=off  OutDataTypeStr=boolean
  Logical Operator12 : Logic  Inputs=8  AllPortsSameDT=off  OutDataTypeStr=boolean
  Logical Operator2 : Logic  Inputs=3  AllPortsSameDT=off  OutDataTypeStr=boolean
  Logical Operator3 : Logic  Inputs=9  AllPortsSameDT=off  OutDataTypeStr=boolean
  Logical Operator4 : Logic  Inputs=10  AllPortsSameDT=off  OutDataTypeStr=boolean
  Logical Operator5 : Logic  AllPortsSameDT=off  OutDataTypeStr=boolean
  Logical Operator6 : Logic  Operator=OR  Inputs=11  AllPortsSameDT=off  OutDataTypeStr=boolean
  Logical Operator7 : Logic  Inputs=11  AllPortsSameDT=off  OutDataTypeStr=boolean
  Logical Operator8 : Logic  Inputs=12  AllPortsSameDT=off  OutDataTypeStr=boolean
  Logical Operator9 : Logic  Inputs=5  AllPortsSameDT=off  OutDataTypeStr=boolean
  Unit Delay{1..11} (11 alike) : UnitDelay  InitialCondition=ModSts.OFFState  SampleTime=-1  HasFrameUpgradeWarning=on
  blockTimeTriggerCond : Outport
  wires:
  Logical Operator8/1 -> Logical Operator6/11
  Logical Operator7/1 -> Logical Operator6/10
  Logical Operator4/1 -> Logical Operator6/9
  Logical Operator3/1 -> Logical Operator6/8
  Logical Operator12/1 -> Logical Operator6/7
  Logical Operator11/1 -> Logical Operator6/6
  Logical Operator10/1 -> Logical Operator6/5
  Logical Operator9/1 -> Logical Operator6/4
  Compare To Constant77/1 -> Logical Operator8/3
  Bus Selector7/2 -> Compare To Constant77/1  "<AEBpartFuncImpArb>"
  Compare To Constant75/1 -> Logical Operator8/5
  Bus Selector7/4 -> Compare To Constant75/1  "<JA_AEBfullFuncImpArb>"
  Compare To Constant73/1 -> Logical Operator8/7
  Bus Selector7/6 -> Compare To Constant73/1  "<JA_PTW_AEBFuncImpArb>"
  Bus Selector7/1 -> Unit Delay7/1, Compare To Constant67/1  "<FCTBFuncImpArb>"
  Compare To Constant71/1 -> Logical Operator8/9
  Bus Selector7/8 -> Compare To Constant71/1  "<JA_pedAEBFuncImpArb>"
  Bus Selector7/11 -> Compare To Constant70/1  "<EBAFuncImpArb>"
  Bus Selector7/10 -> Compare To Constant69/1  "<pedAEBfullFuncImpArb>"
  Compare To Constant70/1 -> Logical Operator8/12
  Compare To Constant69/1 -> Logical Operator8/11
  Compare To Constant68/1 -> Logical Operator8/10
  Compare To Constant67/1 -> Logical Operator8/1
  Unit Delay7/1 -> Compare To Constant66/1
  Bus Selector7/9 -> Compare To Constant68/1  "<cycAEBfullFuncImpArb>"
  Compare To Constant66/1 -> Logical Operator8/2
  Bus Selector7/7 -> Compare To Constant72/1  "<JA_cycAEBFuncImpArb>"
  Compare To Constant72/1 -> Logical Operator8/8
  Bus Selector7/5 -> Compare To Constant74/1  "<AEBfullFuncImpArb>"
  Compare To Constant74/1 -> Logical Operator8/6
  AEBModStsFuncImpArb/1 -> Bus Selector5/1, Bus Selector2/1, Bus Selector1/1, Bus Selector8/1, Bus Selector9/1, Bus Selector11/1, Bus Selector3/1, Bus Selector4/1, Bus Selector6/1, Bus Selector7/1, Bus Selector10/1
  Bus Selector7/3 -> Compare To Constant76/1  "<cycAEBpartFuncImpArb>"
  Compare To Constant76/1 -> Logical Operator8/4
  Compare To Constant43/1 -> Logical Operator7/3
  Bus Selector6/2 -> Compare To Constant43/1  "<cycAEBpartFuncImpArb>"
  Compare To Constant41/1 -> Logical Operator7/5
  Bus Selector6/4 -> Compare To Constant41/1  "<AEBfullFuncImpArb>"
  Compare To Constant39/1 -> Logical Operator7/7
  Bus Selector6/6 -> Compare To Constant39/1  "<JA_cycAEBFuncImpArb>"
  Compare To Constant33/1 -> Logical Operator7/2
  Bus Selector6/8 -> Compare To Constant35/1  "<cycAEBfullFuncImpArb>"
  Unit Delay6/1 -> Compare To Constant33/1
  Compare To Constant34/1 -> Logical Operator7/1
  Compare To Constant35/1 -> Logical Operator7/9
  Compare To Constant36/1 -> Logical Operator7/10
  Compare To Constant37/1 -> Logical Operator7/11
  Bus Selector6/9 -> Compare To Constant36/1  "<pedAEBfullFuncImpArb>"
  Bus Selector6/10 -> Compare To Constant37/1  "<EBAFuncImpArb>"
  Bus Selector6/7 -> Compare To Constant38/1  "<JA_pedAEBFuncImpArb>"
  Compare To Constant38/1 -> Logical Operator7/8
  Bus Selector6/1 -> Unit Delay6/1, Compare To Constant34/1  "<AEBpartFuncImpArb>"
  Bus Selector6/5 -> Compare To Constant40/1  "<JA_PTW_AEBFuncImpArb>"
  Compare To Constant40/1 -> Logical Operator7/6
  Bus Selector6/3 -> Compare To Constant42/1  "<JA_AEBfullFuncImpArb>"
  Compare To Constant42/1 -> Logical Operator7/4
  Compare To Constant32/1 -> Logical Operator4/3
  Bus Selector4/2 -> Compare To Constant32/1  "<JA_AEBfullFuncImpArb>"
  Compare To Constant30/1 -> Logical Operator4/5
  Bus Selector4/4 -> Compare To Constant30/1  "<JA_PTW_AEBFuncImpArb>"
  Bus Selector4/1 -> Unit Delay4/1, Compare To Constant24/1  "<cycAEBpartFuncImpArb>"
  Compare To Constant28/1 -> Logical Operator4/7
  Bus Selector4/6 -> Compare To Constant28/1  "<JA_pedAEBFuncImpArb>"
  Bus Selector4/9 -> Compare To Constant27/1  "<EBAFuncImpArb>"
  Bus Selector4/8 -> Compare To Constant26/1  "<pedAEBfullFuncImpArb>"
  Compare To Constant27/1 -> Logical Operator4/10
  Compare To Constant26/1 -> Logical Operator4/9
  Compare To Constant25/1 -> Logical Operator4/8
  Compare To Constant24/1 -> Logical Operator4/1
  Unit Delay4/1 -> Compare To Constant23/1
  Bus Selector4/7 -> Compare To Constant25/1  "<cycAEBfullFuncImpArb>"
  Compare To Constant23/1 -> Logical Operator4/2
  Bus Selector4/5 -> Compare To Constant29/1  "<JA_cycAEBFuncImpArb>"
  Compare To Constant29/1 -> Logical Operator4/6
  Bus Selector4/3 -> Compare To Constant31/1  "<AEBfullFuncImpArb>"
  Compare To Constant31/1 -> Logical Operator4/4
  Compare To Constant22/1 -> Logical Operator3/3
  Bus Selector3/2 -> Compare To Constant22/1  "<AEBfullFuncImpArb>"
  Compare To Constant20/1 -> Logical Operator3/5
  Bus Selector3/4 -> Compare To Constant20/1  "<JA_cycAEBFuncImpArb>"
  Compare To Constant12/1 -> Logical Operator3/2
  Bus Selector3/6 -> Compare To Constant14/1  "<cycAEBfullFuncImpArb>"
  Unit Delay3/1 -> Compare To Constant12/1
  Compare To Constant13/1 -> Logical Operator3/1
  Compare To Constant14/1 -> Logical Operator3/7
  Compare To Constant15/1 -> Logical Operator3/8
  Compare To Constant18/1 -> Logical Operator3/9
  Bus Selector3/7 -> Compare To Constant15/1  "<pedAEBfullFuncImpArb>"
  Bus Selector3/8 -> Compare To Constant18/1  "<EBAFuncImpArb>"
  Bus Selector3/5 -> Compare To Constant19/1  "<JA_pedAEBFuncImpArb>"
  Compare To Constant19/1 -> Logical Operator3/6
  Bus Selector3/1 -> Compare To Constant13/1, Unit Delay3/1  "<JA_AEBfullFuncImpArb>"
  Bus Selector3/3 -> Compare To Constant21/1  "<JA_PTW_AEBFuncImpArb>"
  Compare To Constant21/1 -> Logical Operator3/4
  Compare To Constant65/1 -> Logical Operator12/3
  Bus Selector11/2 -> Compare To Constant65/1  "<JA_PTW_AEBFuncImpArb>"
  Bus Selector11/1 -> Compare To Constant2/1, Unit Delay11/1  "<AEBfullFuncImpArb>"
  Compare To Constant63/1 -> Logical Operator12/5
  Bus Selector11/4 -> Compare To Constant63/1  "<JA_pedAEBFuncImpArb>"
  Bus Selector11/7 -> Compare To Constant62/1  "<EBAFuncImpArb>"
  Bus Selector11/6 -> Compare To Constant4/1  "<pedAEBfullFuncImpArb>"
  Compare To Constant62/1 -> Logical Operator12/8
  Compare To Constant4/1 -> Logical Operator12/7
  Compare To Constant3/1 -> Logical Operator12/6
  Compare To Constant2/1 -> Logical Operator12/1
  Unit Delay11/1 -> Compare To Constant1/1
  Bus Selector11/5 -> Compare To Constant3/1  "<cycAEBfullFuncImpArb>"
  Compare To Constant1/1 -> Logical Operator12/2
  Bus Selector11/3 -> Compare To Constant64/1  "<JA_cycAEBFuncImpArb>"
  Compare To Constant64/1 -> Logical Operator12/4
  Compare To Constant61/1 -> Logical Operator11/3
  Bus Selector10/2 -> Compare To Constant61/1  "<JA_cycAEBFuncImpArb>"
  Compare To Constant55/1 -> Logical Operator11/2
  Bus Selector10/4 -> Compare To Constant57/1  "<cycAEBfullFuncImpArb>"
  Bus Selector10/1 -> Compare To Constant56/1, Unit Delay10/1  "<JA_PTW_AEBFuncImpArb>"
  Unit Delay10/1 -> Compare To Constant55/1
  Compare To Constant56/1 -> Logical Operator11/1
  Compare To Constant57/1 -> Logical Operator11/5
  Compare To Constant58/1 -> Logical Operator11/6
  Compare To Constant59/1 -> Logical Operator11/7
  Bus Selector10/5 -> Compare To Constant58/1  "<pedAEBfullFuncImpArb>"
  Bus Selector10/6 -> Compare To Constant59/1  "<EBAFuncImpArb>"
  Bus Selector10/3 -> Compare To Constant60/1  "<JA_pedAEBFuncImpArb>"
  Compare To Constant60/1 -> Logical Operator11/4
  Compare To Constant54/1 -> Logical Operator10/3
  Bus Selector9/2 -> Compare To Constant54/1  "<JA_pedAEBFuncImpArb>"
  Bus Selector9/5 -> Compare To Constant53/1  "<EBAFuncImpArb>"
  Bus Selector9/4 -> Compare To Constant52/1  "<pedAEBfullFuncImpArb>"
  Compare To Constant53/1 -> Logical Operator10/6
  Compare To Constant52/1 -> Logical Operator10/5
  Compare To Constant51/1 -> Logical Operator10/4
  Compare To Constant50/1 -> Logical Operator10/1
  Unit Delay9/1 -> Compare To Constant49/1
  Bus Selector9/1 -> Compare To Constant50/1, Unit Delay9/1  "<JA_cycAEBFuncImpArb>"
  Bus Selector9/3 -> Compare To Constant51/1  "<cycAEBfullFuncImpArb>"
  Compare To Constant49/1 -> Logical Operator10/2
  Compare To Constant44/1 -> Logical Operator9/2
  Bus Selector8/2 -> Compare To Constant46/1  "<cycAEBfullFuncImpArb>"
  Bus Selector8/1 -> Compare To Constant45/1, Unit Delay8/1  "<JA_pedAEBFuncImpArb>"
  Unit Delay8/1 -> Compare To Constant44/1
  Compare To Constant45/1 -> Logical Operator9/1
  Compare To Constant46/1 -> Logical Operator9/3
  Compare To Constant47/1 -> Logical Operator9/4
  Compare To Constant48/1 -> Logical Operator9/5
  Bus Selector8/3 -> Compare To Constant47/1  "<pedAEBfullFuncImpArb>"
  Bus Selector8/4 -> Compare To Constant48/1  "<EBAFuncImpArb>"
  Logical Operator1/1 -> Logical Operator6/3
  Logical Operator2/1 -> Logical Operator6/2
  Logical Operator5/1 -> Logical Operator6/1
  Compare To Constant17/1 -> Logical Operator5/1
  Compare To Constant5/1 -> Logical Operator1/2
  Bus Selector1/1 -> Compare To Constant9/1, Unit Delay1/1  "<cycAEBfullFuncImpArb>"
  Unit Delay1/1 -> Compare To Constant5/1
  Compare To Constant6/1 -> Logical Operator1/3
  Logical Operator6/1 -> blockTimeTriggerCond/1  "blockTimeTriggerCond"
  Unit Delay2/1 -> Compare To Constant8/1
  Bus Selector2/1 -> Compare To Constant10/1, Unit Delay2/1  "<pedAEBfullFuncImpArb>"
  Compare To Constant16/1 -> Logical Operator5/2
  Unit Delay5/1 -> Compare To Constant16/1
  Bus Selector5/1 -> Compare To Constant17/1, Unit Delay5/1  "<EBAFuncImpArb>"
  Compare To Constant11/1 -> Logical Operator2/3
  Bus Selector1/2 -> Compare To Constant6/1  "<pedAEBfullFuncImpArb>"
  Compare To Constant9/1 -> Logical Operator1/1
  Compare To Constant7/1 -> Logical Operator1/4
  Bus Selector1/3 -> Compare To Constant7/1  "<EBAFuncImpArb>"
  Compare To Constant10/1 -> Logical Operator2/1
  Compare To Constant8/1 -> Logical Operator2/2
  Bus Selector2/2 -> Compare To Constant11/1  "<EBAFuncImpArb>"

== system TosAeb/FcwAebJa_Step_sys/ALG_AEBSystem/ALG_AEBController/AEB_FuncImpManagementApp/FuncBlockTimeCalc/tBlockTimeBrkReadyCalc/BlockTimeCalc ==
  blockTimeTriggerCond : Inport
  BlockTimeCalc : Stateflow chart  TreatAsAtomicUnit=on
  Constant : Constant  Value=Ts  OutDataTypeStr=single
  Constant1 : Constant  Value=P_AEB_FIM_BrkReadyBlockTimeThres  OutDataTypeStr=single
  blockTime : Outport
  wires:
  Constant1/1 -> BlockTimeCalc/3(PblockTime)
  Constant/1 -> BlockTimeCalc/2(Ts)
  blockTimeTriggerCond/1 -> BlockTimeCalc/1(blockTimeTriggerCond)
  BlockTimeCalc/1(blockTime) -> blockTime/1
